simulink model "test03"  (R2022a) (continued)
== system test03/Subsystem3/Subsystem3/Subsystem1/Subsystem19 ==
  index : Inport
  F : Inport  Port=2
  Add : Sum
  Constant1 : Constant  Value=2
  Constant3 : Constant
  Demux : Demux  Outputs=2
  Subsystem : Subsystem
  Subsystem1 : Subsystem
  Subsystem2 : Subsystem
  Subsystem3 : Subsystem
  Out1 : Outport
  wires:
  Demux/2 -> Subsystem1/3(F)
  Demux/1 -> Subsystem/3(In3)
  F/1 -> Demux/1
  Subsystem2/1(U1) -> Add/2
  Subsystem3/1(U1) -> Add/1
  Add/1 -> Out1/1
  index/1 -> Subsystem1/1(index), Subsystem/1(In1)
  Subsystem/1(Out1) -> Subsystem3/1(uxt1)
  Constant3/1 -> Subsystem/2(k)
  Subsystem/2(tk) -> Subsystem3/2(tk)
  Subsystem1/2(tk) -> Subsystem2/2(tk)
  Subsystem1/1(Out1) -> Subsystem2/1(uxt1)
  Constant1/1 -> Subsystem1/2(k)

== system test03/Subsystem3/Subsystem3/Subsystem1/Subsystem2 ==
  index : Inport
  F : Inport  Port=2
  Add : Sum
  Constant1 : Constant  Value=2
  Constant3 : Constant
  Demux : Demux  Outputs=2
  Subsystem : Subsystem
  Subsystem1 : Subsystem
  Subsystem2 : Subsystem
  Subsystem3 : Subsystem
  Out1 : Outport
  wires:
  Constant1/1 -> Subsystem1/2(k)
  Subsystem1/1(Out1) -> Subsystem2/1(uxt1)
  Subsystem1/2(tk) -> Subsystem2/2(tk)
  Subsystem/2(tk) -> Subsystem3/2(tk)
  Constant3/1 -> Subsystem/2(k)
  Subsystem/1(Out1) -> Subsystem3/1(uxt1)
  index/1 -> Subsystem/1(In1), Subsystem1/1(index)
  Add/1 -> Out1/1
  Subsystem3/1(U1) -> Add/1
  Subsystem2/1(U1) -> Add/2
  F/1 -> Demux/1
  Demux/1 -> Subsystem/3(In3)
  Demux/2 -> Subsystem1/3(F)

== system test03/Subsystem3/Subsystem3/Subsystem1/Subsystem20 ==
  index : Inport
  F : Inport  Port=2
  Add : Sum
  Constant1 : Constant  Value=2
  Constant3 : Constant
  Demux : Demux  Outputs=2
  Subsystem : Subsystem
  Subsystem1 : Subsystem
  Subsystem2 : Subsystem
  Subsystem3 : Subsystem
  Out1 : Outport
  wires:
  Demux/2 -> Subsystem1/3(F)
  Demux/1 -> Subsystem/3(In3)
  F/1 -> Demux/1
  Subsystem2/1(U1) -> Add/2
  Subsystem3/1(U1) -> Add/1
  Add/1 -> Out1/1
  index/1 -> Subsystem1/1(index), Subsystem/1(In1)
  Subsystem/1(Out1) -> Subsystem3/1(uxt1)
  Constant3/1 -> Subsystem/2(k)
  Subsystem/2(tk) -> Subsystem3/2(tk)
  Subsystem1/2(tk) -> Subsystem2/2(tk)
  Subsystem1/1(Out1) -> Subsystem2/1(uxt1)
  Constant1/1 -> Subsystem1/2(k)

== system test03/Subsystem3/Subsystem3/Subsystem1/Subsystem3 ==
  index : Inport
  F : Inport  Port=2
  Add : Sum
  Constant1 : Constant  Value=2
  Constant3 : Constant
  Demux : Demux  Outputs=2
  Subsystem : Subsystem
  Subsystem1 : Subsystem
  Subsystem2 : Subsystem
  Subsystem3 : Subsystem
  Out1 : Outport
  wires:
  Demux/2 -> Subsystem1/3(F)
  Demux/1 -> Subsystem/3(In3)
  F/1 -> Demux/1
  Subsystem2/1(U1) -> Add/2
  Subsystem3/1(U1) -> Add/1
  Add/1 -> Out1/1
  index/1 -> Subsystem1/1(index), Subsystem/1(In1)
  Subsystem/1(Out1) -> Subsystem3/1(uxt1)
  Constant3/1 -> Subsystem/2(k)
  Subsystem/2(tk) -> Subsystem3/2(tk)
  Subsystem1/2(tk) -> Subsystem2/2(tk)
  Subsystem1/1(Out1) -> Subsystem2/1(uxt1)
  Constant1/1 -> Subsystem1/2(k)

== system test03/Subsystem3/Subsystem3/Subsystem1/Subsystem4 ==
  index : Inport
  F : Inport  Port=2
  Add : Sum
  Constant1 : Constant  Value=2
  Constant3 : Constant
  Demux : Demux  Outputs=2
  Subsystem : Subsystem
  Subsystem1 : Subsystem
  Subsystem2 : Subsystem
  Subsystem3 : Subsystem
  Out1 : Outport
  wires:
  Demux/2 -> Subsystem1/3(F)
  Demux/1 -> Subsystem/3(In3)
  F/1 -> Demux/1
  Subsystem2/1(U1) -> Add/2
  Subsystem3/1(U1) -> Add/1
  Add/1 -> Out1/1
  index/1 -> Subsystem1/1(index), Subsystem/1(In1)
  Subsystem/1(Out1) -> Subsystem3/1(uxt1)
  Constant3/1 -> Subsystem/2(k)
  Subsystem/2(tk) -> Subsystem3/2(tk)
  Subsystem1/2(tk) -> Subsystem2/2(tk)
  Subsystem1/1(Out1) -> Subsystem2/1(uxt1)
  Constant1/1 -> Subsystem1/2(k)

== system test03/Subsystem3/Subsystem3/Subsystem1/Subsystem5 ==
  index : Inport
  F : Inport  Port=2
  Add : Sum
  Constant1 : Constant  Value=2
  Constant3 : Constant
  Demux : Demux  Outputs=2
  Subsystem : Subsystem
  Subsystem1 : Subsystem
  Subsystem2 : Subsystem
  Subsystem3 : Subsystem
  Out1 : Outport
  wires:
  Constant1/1 -> Subsystem1/2(k)
  Subsystem1/1(Out1) -> Subsystem2/1(uxt1)
  Subsystem1/2(tk) -> Subsystem2/2(tk)
  Subsystem/2(tk) -> Subsystem3/2(tk)
  Constant3/1 -> Subsystem/2(k)
  Subsystem/1(Out1) -> Subsystem3/1(uxt1)
  index/1 -> Subsystem/1(In1), Subsystem1/1(index)
  Add/1 -> Out1/1
  Subsystem3/1(U1) -> Add/1
  Subsystem2/1(U1) -> Add/2
  F/1 -> Demux/1
  Demux/1 -> Subsystem/3(In3)
  Demux/2 -> Subsystem1/3(F)

== system test03/Subsystem3/Subsystem3/Subsystem1/Subsystem6 ==
  index : Inport
  F : Inport  Port=2
  Add : Sum
  Constant1 : Constant  Value=2
  Constant3 : Constant
  Demux : Demux  Outputs=2
  Subsystem : Subsystem
  Subsystem1 : Subsystem
  Subsystem2 : Subsystem
  Subsystem3 : Subsystem
  Out1 : Outport
  wires:
  Constant1/1 -> Subsystem1/2(k)
  Subsystem1/1(Out1) -> Subsystem2/1(uxt1)
  Subsystem1/2(tk) -> Subsystem2/2(tk)
  Subsystem/2(tk) -> Subsystem3/2(tk)
  Constant3/1 -> Subsystem/2(k)
  Subsystem/1(Out1) -> Subsystem3/1(uxt1)
  index/1 -> Subsystem/1(In1), Subsystem1/1(index)
  Add/1 -> Out1/1
  Subsystem3/1(U1) -> Add/1
  Subsystem2/1(U1) -> Add/2
  F/1 -> Demux/1
  Demux/1 -> Subsystem/3(In3)
  Demux/2 -> Subsystem1/3(F)

== system test03/Subsystem3/Subsystem3/Subsystem1/Subsystem7 ==
  index : Inport
  F : Inport  Port=2
  Add : Sum
  Constant1 : Constant  Value=2
  Constant3 : Constant
  Demux : Demux  Outputs=2
  Subsystem : Subsystem
  Subsystem1 : Subsystem
  Subsystem2 : Subsystem
  Subsystem3 : Subsystem
  Out1 : Outport
  wires:
  Demux/2 -> Subsystem1/3(F)
  Demux/1 -> Subsystem/3(In3)
  F/1 -> Demux/1
  Subsystem2/1(U1) -> Add/2
  Subsystem3/1(U1) -> Add/1
  Add/1 -> Out1/1
  index/1 -> Subsystem1/1(index), Subsystem/1(In1)
  Subsystem/1(Out1) -> Subsystem3/1(uxt1)
  Constant3/1 -> Subsystem/2(k)
  Subsystem/2(tk) -> Subsystem3/2(tk)
  Subsystem1/2(tk) -> Subsystem2/2(tk)
  Subsystem1/1(Out1) -> Subsystem2/1(uxt1)
  Constant1/1 -> Subsystem1/2(k)

== system test03/Subsystem3/Subsystem3/Subsystem1/Subsystem8 ==
  index : Inport
  F : Inport  Port=2
  Add : Sum
  Constant1 : Constant  Value=2
  Constant3 : Constant
  Demux : Demux  Outputs=2
  Subsystem : Subsystem
  Subsystem1 : Subsystem
  Subsystem2 : Subsystem
  Subsystem3 : Subsystem
  Out1 : Outport
  wires:
  Constant1/1 -> Subsystem1/2(k)
  Subsystem1/1(Out1) -> Subsystem2/1(uxt1)
  Subsystem1/2(tk) -> Subsystem2/2(tk)
  Subsystem/2(tk) -> Subsystem3/2(tk)
  Constant3/1 -> Subsystem/2(k)
  Subsystem/1(Out1) -> Subsystem3/1(uxt1)
  index/1 -> Subsystem/1(In1), Subsystem1/1(index)
  Add/1 -> Out1/1
  Subsystem3/1(U1) -> Add/1
  Subsystem2/1(U1) -> Add/2
  F/1 -> Demux/1
  Demux/1 -> Subsystem/3(In3)
  Demux/2 -> Subsystem1/3(F)

== system test03/Subsystem3/Subsystem3/Subsystem1/Subsystem9 ==
  index : Inport
  F : Inport  Port=2
  Add : Sum
  Constant1 : Constant  Value=2
  Constant3 : Constant
  Demux : Demux  Outputs=2
  Subsystem : Subsystem
  Subsystem1 : Subsystem
  Subsystem2 : Subsystem
  Subsystem3 : Subsystem
  Out1 : Outport
  wires:
  Constant1/1 -> Subsystem1/2(k)
  Subsystem1/1(Out1) -> Subsystem2/1(uxt1)
  Subsystem1/2(tk) -> Subsystem2/2(tk)
  Subsystem/2(tk) -> Subsystem3/2(tk)
  Constant3/1 -> Subsystem/2(k)
  Subsystem/1(Out1) -> Subsystem3/1(uxt1)
  index/1 -> Subsystem/1(In1), Subsystem1/1(index)
  Add/1 -> Out1/1
  Subsystem3/1(U1) -> Add/1
  Subsystem2/1(U1) -> Add/2
  F/1 -> Demux/1
  Demux/1 -> Subsystem/3(In3)
  Demux/2 -> Subsystem1/3(F)

== system test03/Subsystem3/Subsystem3/Subsystem2/Subsystem1 ==
  index : Inport
  F : Inport  Port=2
  Add : Sum
  Constant1 : Constant  Value=2
  Constant3 : Constant
  Demux : Demux  Outputs=2
  Subsystem2 : Subsystem
  Subsystem3 : Subsystem
  前轮 : Subsystem
  后轮 : Subsystem
  Out1 : Outport
  wires:
  Constant1/1 -> 后轮/2(k)
  后轮/1(Out1) -> Subsystem2/1(uxt1)
  后轮/2(tk) -> Subsystem2/2(tk)
  前轮/2(tk) -> Subsystem3/2(tk)
  Constant3/1 -> 前轮/2(k)
  前轮/1(Out1) -> Subsystem3/1(uxt1)
  index/1 -> 前轮/1(In1), 后轮/1(index)
  Add/1 -> Out1/1
  Subsystem3/1(U1) -> Add/1
  Subsystem2/1(U1) -> Add/2
  F/1 -> Demux/1
  Demux/1 -> 前轮/3(In3)
  Demux/2 -> 后轮/3(F)

== system test03/Subsystem3/Subsystem3/Subsystem2/Subsystem10 ==
  index : Inport
  F : Inport  Port=2
  Add : Sum
  Constant1 : Constant  Value=2
  Constant3 : Constant
  Demux : Demux  Outputs=2
  Subsystem : Subsystem
  Subsystem1 : Subsystem
  Subsystem2 : Subsystem
  Subsystem3 : Subsystem
  Out1 : Outport
  wires:
  Constant1/1 -> Subsystem1/2(k)
  Subsystem1/1(Out1) -> Subsystem2/1(uxt1)
  Subsystem1/2(tk) -> Subsystem2/2(tk)
  Subsystem/2(tk) -> Subsystem3/2(tk)
  Constant3/1 -> Subsystem/2(k)
  Subsystem/1(Out1) -> Subsystem3/1(uxt1)
  index/1 -> Subsystem/1(In1), Subsystem1/1(index)
  Add/1 -> Out1/1
  Subsystem3/1(U1) -> Add/1
  Subsystem2/1(U1) -> Add/2
  F/1 -> Demux/1
  Demux/1 -> Subsystem/3(In3)
  Demux/2 -> Subsystem1/3(F)

== system test03/Subsystem3/Subsystem3/Subsystem2/Subsystem11 ==
  index : Inport
  F : Inport  Port=2
  Add : Sum
  Constant1 : Constant  Value=2
  Constant3 : Constant
  Demux : Demux  Outputs=2
  Subsystem : Subsystem
  Subsystem1 : Subsystem
  Subsystem2 : Subsystem
  Subsystem3 : Subsystem
  Out1 : Outport
  wires:
  Demux/2 -> Subsystem1/3(F)
  Demux/1 -> Subsystem/3(In3)
  F/1 -> Demux/1
  Subsystem2/1(U1) -> Add/2
  Subsystem3/1(U1) -> Add/1
  Add/1 -> Out1/1
  index/1 -> Subsystem1/1(index), Subsystem/1(In1)
  Subsystem/1(Out1) -> Subsystem3/1(uxt1)
  Constant3/1 -> Subsystem/2(k)
  Subsystem/2(tk) -> Subsystem3/2(tk)
  Subsystem1/2(tk) -> Subsystem2/2(tk)
  Subsystem1/1(Out1) -> Subsystem2/1(uxt1)
  Constant1/1 -> Subsystem1/2(k)

== system test03/Subsystem3/Subsystem3/Subsystem2/Subsystem12 ==
  index : Inport
  F : Inport  Port=2
  Add : Sum
  Constant1 : Constant  Value=2
  Constant3 : Constant
  Demux : Demux  Outputs=2
  Subsystem : Subsystem
  Subsystem1 : Subsystem
  Subsystem2 : Subsystem
  Subsystem3 : Subsystem
  Out1 : Outport
  wires:
  Demux/2 -> Subsystem1/3(F)
  Demux/1 -> Subsystem/3(In3)
  F/1 -> Demux/1
  Subsystem2/1(U1) -> Add/2
  Subsystem3/1(U1) -> Add/1
  Add/1 -> Out1/1
  index/1 -> Subsystem1/1(index), Subsystem/1(In1)
  Subsystem/1(Out1) -> Subsystem3/1(uxt1)
  Constant3/1 -> Subsystem/2(k)
  Subsystem/2(tk) -> Subsystem3/2(tk)
  Subsystem1/2(tk) -> Subsystem2/2(tk)
  Subsystem1/1(Out1) -> Subsystem2/1(uxt1)
  Constant1/1 -> Subsystem1/2(k)

== system test03/Subsystem3/Subsystem3/Subsystem2/Subsystem13 ==
  index : Inport
  F : Inport  Port=2
  Add : Sum
  Constant1 : Constant  Value=2
  Constant3 : Constant
  Demux : Demux  Outputs=2
  Subsystem : Subsystem
  Subsystem1 : Subsystem
  Subsystem2 : Subsystem
  Subsystem3 : Subsystem
  Out1 : Outport
  wires:
  Constant1/1 -> Subsystem1/2(k)
  Subsystem1/1(Out1) -> Subsystem2/1(uxt1)
  Subsystem1/2(tk) -> Subsystem2/2(tk)
  Subsystem/2(tk) -> Subsystem3/2(tk)
  Constant3/1 -> Subsystem/2(k)
  Subsystem/1(Out1) -> Subsystem3/1(uxt1)
  index/1 -> Subsystem/1(In1), Subsystem1/1(index)
  Add/1 -> Out1/1
  Subsystem3/1(U1) -> Add/1
  Subsystem2/1(U1) -> Add/2
  F/1 -> Demux/1
  Demux/1 -> Subsystem/3(In3)
  Demux/2 -> Subsystem1/3(F)

== system test03/Subsystem3/Subsystem3/Subsystem2/Subsystem14 ==
  index : Inport
  F : Inport  Port=2
  Add : Sum
  Constant1 : Constant  Value=2
  Constant3 : Constant
  Demux : Demux  Outputs=2
  Subsystem : Subsystem
  Subsystem1 : Subsystem
  Subsystem2 : Subsystem
  Subsystem3 : Subsystem
  Out1 : Outport
  wires:
  Demux/2 -> Subsystem1/3(F)
  Demux/1 -> Subsystem/3(In3)
  F/1 -> Demux/1
  Subsystem2/1(U1) -> Add/2
  Subsystem3/1(U1) -> Add/1
  Add/1 -> Out1/1
  index/1 -> Subsystem1/1(index), Subsystem/1(In1)
  Subsystem/1(Out1) -> Subsystem3/1(uxt1)
  Constant3/1 -> Subsystem/2(k)
  Subsystem/2(tk) -> Subsystem3/2(tk)
  Subsystem1/2(tk) -> Subsystem2/2(tk)
  Subsystem1/1(Out1) -> Subsystem2/1(uxt1)
  Constant1/1 -> Subsystem1/2(k)

== system test03/Subsystem3/Subsystem3/Subsystem2/Subsystem15 ==
  index : Inport
  F : Inport  Port=2
  Add : Sum
  Constant1 : Constant  Value=2
  Constant3 : Constant
  Demux : Demux  Outputs=2
  Subsystem : Subsystem
  Subsystem1 : Subsystem
  Subsystem2 : Subsystem
  Subsystem3 : Subsystem
  Out1 : Outport
  wires:
  Demux/2 -> Subsystem1/3(F)
  Demux/1 -> Subsystem/3(In3)
  F/1 -> Demux/1
  Subsystem2/1(U1) -> Add/2
  Subsystem3/1(U1) -> Add/1
  Add/1 -> Out1/1
  index/1 -> Subsystem1/1(index), Subsystem/1(In1)
  Subsystem/1(Out1) -> Subsystem3/1(uxt1)
  Constant3/1 -> Subsystem/2(k)
  Subsystem/2(tk) -> Subsystem3/2(tk)
  Subsystem1/2(tk) -> Subsystem2/2(tk)
  Subsystem1/1(Out1) -> Subsystem2/1(uxt1)
  Constant1/1 -> Subsystem1/2(k)

== system test03/Subsystem3/Subsystem3/Subsystem2/Subsystem16 ==
  index : Inport
  F : Inport  Port=2
  Add : Sum
  Constant1 : Constant  Value=2
  Constant3 : Constant
  Demux : Demux  Outputs=2
  Subsystem : Subsystem
  Subsystem1 : Subsystem
  Subsystem2 : Subsystem
  Subsystem3 : Subsystem
  Out1 : Outport
  wires:
  Constant1/1 -> Subsystem1/2(k)
  Subsystem1/1(Out1) -> Subsystem2/1(uxt1)
  Subsystem1/2(tk) -> Subsystem2/2(tk)
  Subsystem/2(tk) -> Subsystem3/2(tk)
  Constant3/1 -> Subsystem/2(k)
  Subsystem/1(Out1) -> Subsystem3/1(uxt1)
  index/1 -> Subsystem/1(In1), Subsystem1/1(index)
  Add/1 -> Out1/1
  Subsystem3/1(U1) -> Add/1
  Subsystem2/1(U1) -> Add/2
  F/1 -> Demux/1
  Demux/1 -> Subsystem/3(In3)
  Demux/2 -> Subsystem1/3(F)

== system test03/Subsystem3/Subsystem3/Subsystem2/Subsystem17 ==
  index : Inport
  F : Inport  Port=2
  Add : Sum
  Constant1 : Constant  Value=2
  Constant3 : Constant
  Demux : Demux  Outputs=2
  Subsystem : Subsystem
  Subsystem1 : Subsystem
  Subsystem2 : Subsystem
  Subsystem3 : Subsystem
  Out1 : Outport
  wires:
  Constant1/1 -> Subsystem1/2(k)
  Subsystem1/1(Out1) -> Subsystem2/1(uxt1)
  Subsystem1/2(tk) -> Subsystem2/2(tk)
  Subsystem/2(tk) -> Subsystem3/2(tk)
  Constant3/1 -> Subsystem/2(k)
  Subsystem/1(Out1) -> Subsystem3/1(uxt1)
  index/1 -> Subsystem/1(In1), Subsystem1/1(index)
  Add/1 -> Out1/1
  Subsystem3/1(U1) -> Add/1
  Subsystem2/1(U1) -> Add/2
  F/1 -> Demux/1
  Demux/1 -> Subsystem/3(In3)
  Demux/2 -> Subsystem1/3(F)

== system test03/Subsystem3/Subsystem3/Subsystem2/Subsystem18 ==
  index : Inport
  F : Inport  Port=2
  Add : Sum
  Constant1 : Constant  Value=2
  Constant3 : Constant
  Demux : Demux  Outputs=2
  Subsystem : Subsystem
  Subsystem1 : Subsystem
  Subsystem2 : Subsystem
  Subsystem3 : Subsystem
  Out1 : Outport
  wires:
  Demux/2 -> Subsystem1/3(F)
  Demux/1 -> Subsystem/3(In3)
  F/1 -> Demux/1
  Subsystem2/1(U1) -> Add/2
  Subsystem3/1(U1) -> Add/1
  Add/1 -> Out1/1
  index/1 -> Subsystem1/1(index), Subsystem/1(In1)
  Subsystem/1(Out1) -> Subsystem3/1(uxt1)
  Constant3/1 -> Subsystem/2(k)
  Subsystem/2(tk) -> Subsystem3/2(tk)
  Subsystem1/2(tk) -> Subsystem2/2(tk)
  Subsystem1/1(Out1) -> Subsystem2/1(uxt1)
  Constant1/1 -> Subsystem1/2(k)

== system test03/Subsystem3/Subsystem3/Subsystem2/Subsystem19 ==
  index : Inport
  F : Inport  Port=2
  Add : Sum
  Constant1 : Constant  Value=2
  Constant3 : Constant
  Demux : Demux  Outputs=2
  Subsystem : Subsystem
  Subsystem1 : Subsystem
  Subsystem2 : Subsystem
  Subsystem3 : Subsystem
  Out1 : Outport
  wires:
  Constant1/1 -> Subsystem1/2(k)
  Subsystem1/1(Out1) -> Subsystem2/1(uxt1)
  Subsystem1/2(tk) -> Subsystem2/2(tk)
  Subsystem/2(tk) -> Subsystem3/2(tk)
  Constant3/1 -> Subsystem/2(k)
  Subsystem/1(Out1) -> Subsystem3/1(uxt1)
  index/1 -> Subsystem/1(In1), Subsystem1/1(index)
  Add/1 -> Out1/1
  Subsystem3/1(U1) -> Add/1
  Subsystem2/1(U1) -> Add/2
  F/1 -> Demux/1
  Demux/1 -> Subsystem/3(In3)
  Demux/2 -> Subsystem1/3(F)

== system test03/Subsystem3/Subsystem3/Subsystem2/Subsystem2 ==
  index : Inport
  F : Inport  Port=2
  Add : Sum
  Constant1 : Constant  Value=2
  Constant3 : Constant
  Demux : Demux  Outputs=2
  Subsystem : Subsystem
  Subsystem1 : Subsystem
  Subsystem2 : Subsystem
  Subsystem3 : Subsystem
  Out1 : Outport
  wires:
  Demux/2 -> Subsystem1/3(F)
  Demux/1 -> Subsystem/3(In3)
  F/1 -> Demux/1
  Subsystem2/1(U1) -> Add/2
  Subsystem3/1(U1) -> Add/1
  Add/1 -> Out1/1
  index/1 -> Subsystem1/1(index), Subsystem/1(In1)
  Subsystem/1(Out1) -> Subsystem3/1(uxt1)
  Constant3/1 -> Subsystem/2(k)
  Subsystem/2(tk) -> Subsystem3/2(tk)
  Subsystem1/2(tk) -> Subsystem2/2(tk)
  Subsystem1/1(Out1) -> Subsystem2/1(uxt1)
  Constant1/1 -> Subsystem1/2(k)

== system test03/Subsystem3/Subsystem3/Subsystem2/Subsystem20 ==
  index : Inport
  F : Inport  Port=2
  Add : Sum
  Constant1 : Constant  Value=2
  Constant3 : Constant
  Demux : Demux  Outputs=2
  Subsystem : Subsystem
  Subsystem1 : Subsystem
  Subsystem2 : Subsystem
  Subsystem3 : Subsystem
  Out1 : Outport
  wires:
  Constant1/1 -> Subsystem1/2(k)
  Subsystem1/1(Out1) -> Subsystem2/1(uxt1)
  Subsystem1/2(tk) -> Subsystem2/2(tk)
  Subsystem/2(tk) -> Subsystem3/2(tk)
  Constant3/1 -> Subsystem/2(k)
  Subsystem/1(Out1) -> Subsystem3/1(uxt1)
  index/1 -> Subsystem/1(In1), Subsystem1/1(index)
  Add/1 -> Out1/1
  Subsystem3/1(U1) -> Add/1
  Subsystem2/1(U1) -> Add/2
  F/1 -> Demux/1
  Demux/1 -> Subsystem/3(In3)
  Demux/2 -> Subsystem1/3(F)

== system test03/Subsystem3/Subsystem3/Subsystem2/Subsystem3 ==
  index : Inport
  F : Inport  Port=2
  Add : Sum
  Constant1 : Constant  Value=2
  Constant3 : Constant
  Demux : Demux  Outputs=2
  Subsystem : Subsystem
  Subsystem1 : Subsystem
  Subsystem2 : Subsystem
  Subsystem3 : Subsystem
  Out1 : Outport
  wires:
  Constant1/1 -> Subsystem1/2(k)
  Subsystem1/1(Out1) -> Subsystem2/1(uxt1)
  Subsystem1/2(tk) -> Subsystem2/2(tk)
  Subsystem/2(tk) -> Subsystem3/2(tk)
  Constant3/1 -> Subsystem/2(k)
  Subsystem/1(Out1) -> Subsystem3/1(uxt1)
  index/1 -> Subsystem/1(In1), Subsystem1/1(index)
  Add/1 -> Out1/1
  Subsystem3/1(U1) -> Add/1
  Subsystem2/1(U1) -> Add/2
  F/1 -> Demux/1
  Demux/1 -> Subsystem/3(In3)
  Demux/2 -> Subsystem1/3(F)

== system test03/Subsystem3/Subsystem3/Subsystem2/Subsystem4 ==
  index : Inport
  F : Inport  Port=2
  Add : Sum
  Constant1 : Constant  Value=2
  Constant3 : Constant
  Demux : Demux  Outputs=2
  Subsystem : Subsystem
  Subsystem1 : Subsystem
  Subsystem2 : Subsystem
  Subsystem3 : Subsystem
  Out1 : Outport
  wires:
  Constant1/1 -> Subsystem1/2(k)
  Subsystem1/1(Out1) -> Subsystem2/1(uxt1)
  Subsystem1/2(tk) -> Subsystem2/2(tk)
  Subsystem/2(tk) -> Subsystem3/2(tk)
  Constant3/1 -> Subsystem/2(k)
  Subsystem/1(Out1) -> Subsystem3/1(uxt1)
  index/1 -> Subsystem/1(In1), Subsystem1/1(index)
  Add/1 -> Out1/1
  Subsystem3/1(U1) -> Add/1
  Subsystem2/1(U1) -> Add/2
  F/1 -> Demux/1
  Demux/1 -> Subsystem/3(In3)
  Demux/2 -> Subsystem1/3(F)

== system test03/Subsystem3/Subsystem3/Subsystem2/Subsystem5 ==
  index : Inport
  F : Inport  Port=2
  Add : Sum
  Constant1 : Constant  Value=2
  Constant3 : Constant
  Demux : Demux  Outputs=2
  Subsystem : Subsystem
  Subsystem1 : Subsystem
  Subsystem2 : Subsystem
  Subsystem3 : Subsystem
  Out1 : Outport
  wires:
  Demux/2 -> Subsystem1/3(F)
  Demux/1 -> Subsystem/3(In3)
  F/1 -> Demux/1
  Subsystem2/1(U1) -> Add/2
  Subsystem3/1(U1) -> Add/1
  Add/1 -> Out1/1
  index/1 -> Subsystem1/1(index), Subsystem/1(In1)
  Subsystem/1(Out1) -> Subsystem3/1(uxt1)
  Constant3/1 -> Subsystem/2(k)
  Subsystem/2(tk) -> Subsystem3/2(tk)
  Subsystem1/2(tk) -> Subsystem2/2(tk)
  Subsystem1/1(Out1) -> Subsystem2/1(uxt1)
  Constant1/1 -> Subsystem1/2(k)

== system test03/Subsystem3/Subsystem3/Subsystem2/Subsystem6 ==
  index : Inport
  F : Inport  Port=2
  Add : Sum
  Constant1 : Constant  Value=2
  Constant3 : Constant
  Demux : Demux  Outputs=2
  Subsystem : Subsystem
  Subsystem1 : Subsystem
  Subsystem2 : Subsystem
  Subsystem3 : Subsystem
  Out1 : Outport
  wires:
  Demux/2 -> Subsystem1/3(F)
  Demux/1 -> Subsystem/3(In3)
  F/1 -> Demux/1
  Subsystem2/1(U1) -> Add/2
  Subsystem3/1(U1) -> Add/1
  Add/1 -> Out1/1
  index/1 -> Subsystem1/1(index), Subsystem/1(In1)
  Subsystem/1(Out1) -> Subsystem3/1(uxt1)
  Constant3/1 -> Subsystem/2(k)
  Subsystem/2(tk) -> Subsystem3/2(tk)
  Subsystem1/2(tk) -> Subsystem2/2(tk)
  Subsystem1/1(Out1) -> Subsystem2/1(uxt1)
  Constant1/1 -> Subsystem1/2(k)

== system test03/Subsystem3/Subsystem3/Subsystem2/Subsystem7 ==
  index : Inport
  F : Inport  Port=2
  Add : Sum
  Constant1 : Constant  Value=2
  Constant3 : Constant
  Demux : Demux  Outputs=2
  Subsystem : Subsystem
  Subsystem1 : Subsystem
  Subsystem2 : Subsystem
  Subsystem3 : Subsystem
  Out1 : Outport
  wires:
  Constant1/1 -> Subsystem1/2(k)
  Subsystem1/1(Out1) -> Subsystem2/1(uxt1)
  Subsystem1/2(tk) -> Subsystem2/2(tk)
  Subsystem/2(tk) -> Subsystem3/2(tk)
  Constant3/1 -> Subsystem/2(k)
  Subsystem/1(Out1) -> Subsystem3/1(uxt1)
  index/1 -> Subsystem/1(In1), Subsystem1/1(index)
  Add/1 -> Out1/1
  Subsystem3/1(U1) -> Add/1
  Subsystem2/1(U1) -> Add/2
  F/1 -> Demux/1
  Demux/1 -> Subsystem/3(In3)
  Demux/2 -> Subsystem1/3(F)

== system test03/Subsystem3/Subsystem3/Subsystem2/Subsystem8 ==
  index : Inport
  F : Inport  Port=2
  Add : Sum
  Constant1 : Constant  Value=2
  Constant3 : Constant
  Demux : Demux  Outputs=2
  Subsystem : Subsystem
  Subsystem1 : Subsystem
  Subsystem2 : Subsystem
  Subsystem3 : Subsystem
  Out1 : Outport
  wires:
  Demux/2 -> Subsystem1/3(F)
  Demux/1 -> Subsystem/3(In3)
  F/1 -> Demux/1
  Subsystem2/1(U1) -> Add/2
  Subsystem3/1(U1) -> Add/1
  Add/1 -> Out1/1
  index/1 -> Subsystem1/1(index), Subsystem/1(In1)
  Subsystem/1(Out1) -> Subsystem3/1(uxt1)
  Constant3/1 -> Subsystem/2(k)
  Subsystem/2(tk) -> Subsystem3/2(tk)
  Subsystem1/2(tk) -> Subsystem2/2(tk)
  Subsystem1/1(Out1) -> Subsystem2/1(uxt1)
  Constant1/1 -> Subsystem1/2(k)

== system test03/Subsystem3/Subsystem3/Subsystem2/Subsystem9 ==
  index : Inport
  F : Inport  Port=2
  Add : Sum
  Constant1 : Constant  Value=2
  Constant3 : Constant
  Demux : Demux  Outputs=2
  Subsystem : Subsystem
  Subsystem1 : Subsystem
  Subsystem2 : Subsystem
  Subsystem3 : Subsystem
  Out1 : Outport
  wires:
  Demux/2 -> Subsystem1/3(F)
  Demux/1 -> Subsystem/3(In3)
  F/1 -> Demux/1
  Subsystem2/1(U1) -> Add/2
  Subsystem3/1(U1) -> Add/1
  Add/1 -> Out1/1
  index/1 -> Subsystem1/1(index), Subsystem/1(In1)
  Subsystem/1(Out1) -> Subsystem3/1(uxt1)
  Constant3/1 -> Subsystem/2(k)
  Subsystem/2(tk) -> Subsystem3/2(tk)
  Subsystem1/2(tk) -> Subsystem2/2(tk)
  Subsystem1/1(Out1) -> Subsystem2/1(uxt1)
  Constant1/1 -> Subsystem1/2(k)

== system test03/Subsystem3/Subsystem3/Subsystem3/Subsystem1 ==
  index : Inport
  F : Inport  Port=2
  Add : Sum
  Constant1 : Constant  Value=2
  Constant3 : Constant
  Demux : Demux  Outputs=2
  Subsystem2 : Subsystem
  Subsystem3 : Subsystem
  前轮 : Subsystem
  后轮 : Subsystem
  Out1 : Outport
  wires:
  Demux/2 -> 后轮/3(F)
  Demux/1 -> 前轮/3(In3)
  F/1 -> Demux/1
  Subsystem2/1(U1) -> Add/2
  Subsystem3/1(U1) -> Add/1
  Add/1 -> Out1/1
  index/1 -> 后轮/1(index), 前轮/1(In1)
  前轮/1(Out1) -> Subsystem3/1(uxt1)
  Constant3/1 -> 前轮/2(k)
  前轮/2(tk) -> Subsystem3/2(tk)
  后轮/2(tk) -> Subsystem2/2(tk)
  后轮/1(Out1) -> Subsystem2/1(uxt1)
  Constant1/1 -> 后轮/2(k)

== system test03/Subsystem3/Subsystem3/Subsystem3/Subsystem10 ==
  index : Inport
  F : Inport  Port=2
  Add : Sum
  Constant1 : Constant  Value=2
  Constant3 : Constant
  Demux : Demux  Outputs=2
  Subsystem : Subsystem
  Subsystem1 : Subsystem
  Subsystem2 : Subsystem
  Subsystem3 : Subsystem
  Out1 : Outport
  wires:
  Demux/2 -> Subsystem1/3(F)
  Demux/1 -> Subsystem/3(In3)
  F/1 -> Demux/1
  Subsystem2/1(U1) -> Add/2
  Subsystem3/1(U1) -> Add/1
  Add/1 -> Out1/1
  index/1 -> Subsystem1/1(index), Subsystem/1(In1)
  Subsystem/1(Out1) -> Subsystem3/1(uxt1)
  Constant3/1 -> Subsystem/2(k)
  Subsystem/2(tk) -> Subsystem3/2(tk)
  Subsystem1/2(tk) -> Subsystem2/2(tk)
  Subsystem1/1(Out1) -> Subsystem2/1(uxt1)
  Constant1/1 -> Subsystem1/2(k)

== system test03/Subsystem3/Subsystem3/Subsystem3/Subsystem11 ==
  index : Inport
  F : Inport  Port=2
  Add : Sum
  Constant1 : Constant  Value=2
  Constant3 : Constant
  Demux : Demux  Outputs=2
  Subsystem : Subsystem
  Subsystem1 : Subsystem
  Subsystem2 : Subsystem
  Subsystem3 : Subsystem
  Out1 : Outport
  wires:
  Constant1/1 -> Subsystem1/2(k)
  Subsystem1/1(Out1) -> Subsystem2/1(uxt1)
  Subsystem1/2(tk) -> Subsystem2/2(tk)
  Subsystem/2(tk) -> Subsystem3/2(tk)
  Constant3/1 -> Subsystem/2(k)
  Subsystem/1(Out1) -> Subsystem3/1(uxt1)
  index/1 -> Subsystem/1(In1), Subsystem1/1(index)
  Add/1 -> Out1/1
  Subsystem3/1(U1) -> Add/1
  Subsystem2/1(U1) -> Add/2
  F/1 -> Demux/1
  Demux/1 -> Subsystem/3(In3)
  Demux/2 -> Subsystem1/3(F)

== system test03/Subsystem3/Subsystem3/Subsystem3/Subsystem12 ==
  index : Inport
  F : Inport  Port=2
  Add : Sum
  Constant1 : Constant  Value=2
  Constant3 : Constant
  Demux : Demux  Outputs=2
  Subsystem : Subsystem
  Subsystem1 : Subsystem
  Subsystem2 : Subsystem
  Subsystem3 : Subsystem
  Out1 : Outport
  wires:
  Constant1/1 -> Subsystem1/2(k)
  Subsystem1/1(Out1) -> Subsystem2/1(uxt1)
  Subsystem1/2(tk) -> Subsystem2/2(tk)
  Subsystem/2(tk) -> Subsystem3/2(tk)
  Constant3/1 -> Subsystem/2(k)
  Subsystem/1(Out1) -> Subsystem3/1(uxt1)
  index/1 -> Subsystem/1(In1), Subsystem1/1(index)
  Add/1 -> Out1/1
  Subsystem3/1(U1) -> Add/1
  Subsystem2/1(U1) -> Add/2
  F/1 -> Demux/1
  Demux/1 -> Subsystem/3(In3)
  Demux/2 -> Subsystem1/3(F)
